simulink model "TosAeb"  (R2021a)
settings: Solver=FixedStepDiscrete  StartTime=0.0  StopTime=inf  FixedStep=0.01  RelTol=1e-3
undefined symbols (not defined in the file or in its folder): ACCModSts, AccSts1, AEB_ObjectMovingOrientation, AEB_ObjectMovingState, CN_BTCActive, CN_CurrentGearR, CN_CurrentGearR_DHT, CN_PTCActive, CN_VDCActive, CN_VSC_EspOff, CN_VSC_FCW_AEB_Error, CN_VSC_FCW_AEB_NoDispay, CN_VSC_FCW_AEB_PerfmDgdtn, CN_VSC_FCW_AEB_TempUnAvlbl, CN_VSC_VehStandstill, CNaccelAvoidMax, CNaccelAvoidMin, CNbrakePedalPress, CNcomfortStrLeftAccelMax, CNcomfortStrLeftAccelMin, CNcomfortStrRightAccelMax, CNcomfortStrRightAccelMin, CNDrvDoorOpen, CNDrvModeEco, CNDrvModeNorm, CNDrvModeOther, CNDrvModeSport, CNDrvSBRreminder, CNNull, CNobjInvalidID, CNPassDoorOpen, CNPassSBRreminder, CNPEBSAccelNegInf, CNPEBSAccelNull, CNPEBSTimeInf, CNPEBSTimeNull, CNSTOPMAX, CNSTOPNULL, CNsubjWidth, CNSwtOFF, CNSwtON, CNTTCMAX, CNTTCNULL, CNVehConfigAEBCycNone, CNVehConfigAEBNone, CNVehConfigAEBPedNone, CNVehConfigFCWNone, CNVehConfigJANone, CollisionSeverity, FALSE, FCWSnvty, JACycObjCalcInfoDefaultParams, JAPedObjCalcInfoDefaultParams, JAVehObjCalcInfoDefaultParams, latCycObjCalcInfoDefaultParams, latPedObjCalcInfoDefaultParams, longObjCalcInfoDefaultParams, ModSts, movingStatus, ObjectType ... (+1112)
libraries: simulink x3031, AEBSFunctionLib x50, Ta_Library x33, AEBSStateMachineLib x23, Mylibrary x12, simulink_extras x7, ObjSelect_lib x3, dspstat3 x2
(part 73 of 102)

== system TosAeb/FcwAebJa_Step_sys/ALG_AEBSystem/ALG_AEBController/AEB_LongTgtInfoCalc/LongObjCalcInfoCalc/AEBS_LongCollisionAssesApp/TTCCalc/TTCCalc/Situation2/distCorrCalc/AccelEqualZeroJudge ==
  subjLongAccel : Inport
  Abs : Abs
  Compare To Constant : simulink/Logic and Bit Operations/Compare To Constant  relop=<=  const=0.1  OutDataTypeStr=boolean
  accelEqualZeroFlg : Outport
  wires:
  subjLongAccel/1 -> Abs/1
  Compare To Constant/1 -> accelEqualZeroFlg/1
  Abs/1 -> Compare To Constant/1

== system TosAeb/FcwAebJa_Step_sys/ALG_AEBSystem/ALG_AEBController/AEB_LongTgtInfoCalc/LongObjCalcInfoCalc/AEBS_LongCollisionAssesApp/TTCCalc/TTCCalc/Situation2/distCorrCalc/distCorrCalc ==
  distEgo : Inport
  dxFrontBumper : Inport  Port=2
  Add : Sum
  distCorr : Outport
  wires:
  distEgo/1 -> Add/1
  dxFrontBumper/1 -> Add/2
  Add/1 -> distCorr/1

== system TosAeb/FcwAebJa_Step_sys/ALG_AEBSystem/ALG_AEBController/AEB_LongTgtInfoCalc/LongObjCalcInfoCalc/AEBS_LongCollisionAssesApp/TTCCalc/TTCCalc/Situation2/distCorrCalc/subjLongAccelEqualZero ==
  Action Port : ActionPort  ActionPortLabel=if(u1)
  Constant : Constant  Value=CNNull
  distEgo : Outport
  wires:
  Constant/1 -> distEgo/1

== system TosAeb/FcwAebJa_Step_sys/ALG_AEBSystem/ALG_AEBController/AEB_LongTgtInfoCalc/LongObjCalcInfoCalc/AEBS_LongCollisionAssesApp/TTCCalc/TTCCalc/Situation2/distCorrCalc/subjLongAccelNoEqualZero ==
  subjVel : Inport
  subjLongAccel : Inport  Port=2
  Action Port : ActionPort  ActionPortLabel=else
  Divide : Product  Inputs=*/
  Gain : Gain  Gain=0.5
  Math Function : Math  Operator=square  SignedPower=on  SaturateOnIntegerOverflow=on
  distEgo : Outport
  wires:
  subjVel/1 -> Math Function/1
  Math Function/1 -> Divide/1
  subjLongAccel/1 -> Divide/2
  Divide/1 -> Gain/1
  Gain/1 -> distEgo/1

== system TosAeb/FcwAebJa_Step_sys/ALG_AEBSystem/ALG_AEBController/AEB_LongTgtInfoCalc/LongObjCalcInfoCalc/AEBS_LongCollisionAssesApp/TTCCalc/TTCCalc/Situation3/SloveTTCQuadraticEquation/AccelRelEqualsZero ==
  distRel : Inport
  velRel : Inport  Port=2
  AccelRelEqualZero : ActionPort  ActionPortLabel=if(u1)
  Chart : Stateflow chart  TreatAsAtomicUnit=on
  TTC : Outport
  wires:
  Chart/1(TTC) -> TTC/1  "TTC"
  distRel/1 -> Chart/1(distRel)  "distRel"
  velRel/1 -> Chart/2(velRel)  "velRel"

== system TosAeb/FcwAebJa_Step_sys/ALG_AEBSystem/ALG_AEBController/AEB_LongTgtInfoCalc/LongObjCalcInfoCalc/AEBS_LongCollisionAssesApp/TTCCalc/TTCCalc/Situation3/SloveTTCQuadraticEquation/AccelRelUnequalZero ==
  accelRel : Inport
  velRel : Inport  Port=2
  distRel : Inport  Port=3
  AccelRelUnequalZero : ActionPort  ActionPortLabel=else
  DeltaCalcu : Subsystem
  DeltaGreaterEqualZero : Subsystem  TreatAsAtomicUnit=on
  DeltaSmallerZero : Subsystem  TreatAsAtomicUnit=on
  JudeDelta : If  IfExpression=u1 >= 0
  Merge : Merge
  TTC : Outport
  wires:
  Merge/1 -> TTC/1  "TTC"
  DeltaCalcu/1(delta) -> JudeDelta/1, DeltaGreaterEqualZero/1(delta)  "delta"
  accelRel/1 -> DeltaCalcu/1(accelRel), DeltaGreaterEqualZero/3(accelRel)  "accelRel"
  velRel/1 -> DeltaCalcu/2(velRel), DeltaGreaterEqualZero/2(velRel)  "velRel"
  distRel/1 -> DeltaCalcu/3(distRel)  "distRel"
  DeltaSmallerZero/1(TTC) -> Merge/2
  DeltaGreaterEqualZero/1(TTC) -> Merge/1
  JudeDelta/1 -> DeltaGreaterEqualZero/Action
  JudeDelta/2 -> [DeltaSmallZero]
  [DeltaSmallZero] -> DeltaSmallerZero/Action

== system TosAeb/FcwAebJa_Step_sys/ALG_AEBSystem/ALG_AEBController/AEB_LongTgtInfoCalc/LongObjCalcInfoCalc/AEBS_LongCollisionAssesApp/TTCCalc/TTCCalc/Situation3/SloveTTCQuadraticEquation/accelZeroFlgCalc ==
  accel : Inport
  Abs : Abs
  P_LongCA_AccelObjFiltThesTTC : simulink/Logic and Bit Operations/Compare To Constant  relop=<=  const=P_AEB_LongCA_AccelObjFiltThesTTC  OutDataTypeStr=boolean
  accelZeroFlg : Outport
  wires:
  Abs/1 -> P_LongCA_AccelObjFiltThesTTC/1
  P_LongCA_AccelObjFiltThesTTC/1 -> accelZeroFlg/1  "accelZeroFlg"
  accel/1 -> Abs/1  "accel"

== system TosAeb/FcwAebJa_Step_sys/ALG_AEBSystem/ALG_AEBController/AEB_LongTgtInfoCalc/LongObjCalcInfoCalc/AEBS_LongCollisionAssesApp/TTCCalc/TTCCalc/Situation3/distCorrCalc/AccelZeroJudge ==
  axAbsObj : Inport
  Abs : Abs
  Compare To Constant : simulink/Logic and Bit Operations/Compare To Constant  relop=<=  const=0.1  OutDataTypeStr=boolean
  accelZeroFlg : Outport
  wires:
  axAbsObj/1 -> Abs/1
  Compare To Constant/1 -> accelZeroFlg/1
  Abs/1 -> Compare To Constant/1

== system TosAeb/FcwAebJa_Step_sys/ALG_AEBSystem/ALG_AEBController/AEB_LongTgtInfoCalc/LongObjCalcInfoCalc/AEBS_LongCollisionAssesApp/TTCCalc/TTCCalc/Situation3/distCorrCalc/AxObjEqualZero ==
  Action Port : ActionPort  ActionPortLabel=if(u1)
  Constant : Constant  Value=CNNull
  distObj : Outport
  wires:
  Constant/1 -> distObj/1

== system TosAeb/FcwAebJa_Step_sys/ALG_AEBSystem/ALG_AEBController/AEB_LongTgtInfoCalc/LongObjCalcInfoCalc/AEBS_LongCollisionAssesApp/TTCCalc/TTCCalc/Situation3/distCorrCalc/AxObjUnequalZero ==
  vxAbsObj : Inport
  axAbsObj : Inport  Port=2
  Action Port : ActionPort  ActionPortLabel=else
  Divide : Product  Inputs=*/
  Gain : Gain  Gain=0.5
  Math Function : Math  Operator=square  SignedPower=on  SaturateOnIntegerOverflow=on
  distObj : Outport
  wires:
  Divide/1 -> Gain/1
  axAbsObj/1 -> Divide/2
  Math Function/1 -> Divide/1
  vxAbsObj/1 -> Math Function/1
  Gain/1 -> distObj/1

== system TosAeb/FcwAebJa_Step_sys/ALG_AEBSystem/ALG_AEBController/AEB_LongTgtInfoCalc/LongObjCalcInfoCalc/AEBS_LongCollisionAssesApp/TTCCalc/TTCCalc/Situation3/distCorrCalc/distCorrCalc ==
  dxFrontBumper : Inport
  distObj : Inport  Port=2
  Add : Sum  Inputs=+-
  distCorr : Outport
  wires:
  Add/1 -> distCorr/1
  dxFrontBumper/1 -> Add/1
  distObj/1 -> Add/2

== system TosAeb/FcwAebJa_Step_sys/ALG_AEBSystem/ALG_AEBController/AEB_LongTgtInfoCalc/LongObjCalcInfoCalc/AEBS_LongCollisionAssesApp/TTCCalc/TTCCalc/SloveTTCQuadraticEquation/AccelRelEqualsZero/Chart/TTCCalc ==
  distRel : Inport
  velRel : Inport  Port=2
  TTCCalc : TriggerPort  TriggerType=function-call  VariantControl=Variant
      settings: CopyFcn=Stateflow.SLINSF.SimulinkMan.removeBlockPreDeleteFcn  PreDeleteFcn=Stateflow.SLINSF.SimulinkMan.unsafePredeleteFcn
  Abs : Abs
  Abs1 : Abs
  Divide : Product  Inputs=*/
  tempTTC : Outport
  wires:
  Abs1/1 -> Divide/1
  Divide/1 -> tempTTC/1  "TTC"
  velRel/1 -> Abs/1  "velRel"
  distRel/1 -> Abs1/1  "distRel"
  Abs/1 -> Divide/2

== system TosAeb/FcwAebJa_Step_sys/ALG_AEBSystem/ALG_AEBController/AEB_LongTgtInfoCalc/LongObjCalcInfoCalc/AEBS_LongCollisionAssesApp/TTCCalc/TTCCalc/SloveTTCQuadraticEquation/AccelRelUnequalZero/DeltaCalcu ==
  accelRel : Inport
  velRel : Inport  Port=2
  distRel : Inport  Port=3
  Gain : Gain  Gain=2
  Math Function : Math  Operator=square  SignedPower=on  SaturateOnIntegerOverflow=on
  Product : Product
  Subtract : Sum  Inputs=+-
  delta : Outport
  wires:
  distRel/1 -> Product/2  "distRel"
  Math Function/1 -> Subtract/1
  Subtract/1 -> delta/1  "delta"
  Gain/1 -> Subtract/2
  Product/1 -> Gain/1
  velRel/1 -> Math Function/1  "velRel"
  accelRel/1 -> Product/1  "accelRel"

== system TosAeb/FcwAebJa_Step_sys/ALG_AEBSystem/ALG_AEBController/AEB_LongTgtInfoCalc/LongObjCalcInfoCalc/AEBS_LongCollisionAssesApp/TTCCalc/TTCCalc/SloveTTCQuadraticEquation/AccelRelUnequalZero/DeltaGreaterEqualZero ==
  delta : Inport
  velRel : Inport  Port=2
  accelRel : Inport  Port=3
  DeltaGreaterEqualZero : ActionPort  ActionPortLabel=if(u1 >= 0)
  Divide : Product  Inputs=*/
  Divide1 : Product  Inputs=*/
  Gain1 : Gain  Gain=-1
  RawTTCCalcu : Stateflow chart  TreatAsAtomicUnit=on
  Sqrt : Sqrt  SaturateOnIntegerOverflow=on
  Subtract1 : Sum  Inputs=+-
  Subtract2 : Sum
  TTC : Outport
  wires:
  Subtract1/1 -> Divide/1
  velRel/1 -> Gain1/1  "velRel"
  Subtract2/1 -> Divide1/1
  delta/1 -> Sqrt/1  "delta"
  Gain1/1 -> Subtract2/2, Subtract1/1
  accelRel/1 -> Divide/2, Divide1/2  "accelRel"
  Divide/1 -> RawTTCCalcu/1(TTC1)  "TTC1"
  Divide1/1 -> RawTTCCalcu/2(TTC2)  "TTC2"
  RawTTCCalcu/1(TTC) -> TTC/1  "TTC"
  Sqrt/1 -> Subtract2/1, Subtract1/2

== system TosAeb/FcwAebJa_Step_sys/ALG_AEBSystem/ALG_AEBController/AEB_LongTgtInfoCalc/LongObjCalcInfoCalc/AEBS_LongCollisionAssesApp/TTCCalc/TTCCalc/SloveTTCQuadraticEquation/AccelRelUnequalZero/DeltaSmallerZero ==
  DeltaSmallerZero : ActionPort  ActionPortLabel=else
  Constant : Constant  Value=CNTTCMAX
  TTC : Outport
  wires:
  Constant/1 -> TTC/1  "TTC"

== system TosAeb/FcwAebJa_Step_sys/ALG_AEBSystem/ALG_AEBController/AEB_LongTgtInfoCalc/LongObjCalcInfoCalc/AEBS_LongCollisionAssesApp/collisionSeverityHighCalc/collisionSeverityHighCalc1/CollisionAssessHighCalcVersion3/CollisionAssessHighCalc1/CalcBrakePhase1 ==
  a0Ego : Inport
  a0Obj : Inport  Port=2
  tBrakeLatency : Inport  Port=3
  velMaxReudce : Inport  Port=4
  v0Ego : Inport  Port=5
  v0Obj : Inport  Port=6
  dx : Inport  Port=7
  Bus Creator : BusCreator  Inputs=7
  Bus Selector : BusSelector  OutputSignals=a0Ego,v0Ego
  Bus Selector1 : BusSelector  OutputSignals=v0Ego,velMaxReudce,a0Ego,tBrakeLatency
  Bus Selector2 : BusSelector  OutputSignals=a0Obj,v0Obj
  Bus Selector3 : BusSelector  OutputSignals=v0Ego,a0Ego
  Bus Selector4 : BusSelector  OutputSignals=v0Obj,a0Obj
  Bus Selector5 : BusSelector  OutputSignals=v0Ego,a0Ego
  Bus Selector6 : BusSelector  OutputSignals=a0Obj,v0Obj
  Bus Selector7 : BusSelector  OutputSignals=dx
  Bus Selector8 : BusSelector  OutputSignals=velMaxReudce,v0Ego
  egoCollisionFlgCalc : Subsystem
  restDeltaVelCalc : Subsystem
  stEgoCalc : Subsystem
  stObjCalc : Subsystem
  tEgoStopCalc : Subsystem
  tObjRunCalc : Subsystem
  tObjStopCalc : Subsystem
  time4EgoPredic : Subsystem
  timeEgovDeltaCalc : Subsystem
  vtEgoCalc : Subsystem
  vtObjCalc : Subsystem
  egoCollisionFlg : Outport
  restDeltaVel : Outport  Port=2
  vtEgo : Outport  Port=3
  vtObj : Outport  Port=4
  dxt : Outport  Port=5
  wires:
  Bus Selector2/1 -> tObjStopCalc/1(a0Obj)  "<a0Obj>"
  Bus Selector2/2 -> tObjStopCalc/2(v0Obj)  "<v0Obj>"
  tEgoStopCalc/1(tEgoStop) -> time4EgoPredic/1(tEgoStop)
  Bus Selector/1 -> tEgoStopCalc/1(a0Ego)  "<a0Ego>"
  Bus Selector/2 -> tEgoStopCalc/2(v0Ego)  "<v0Ego>"
  Bus Creator/1 -> [BrakePhaseInput], Bus Selector2/1, Bus Selector1/1, Bus Selector/1, Bus Selector8/1, Bus Selector3/1
  Bus Selector6/2 -> stObjCalc/3(v0)  "<v0Obj>"
  Bus Selector6/1 -> stObjCalc/2(a0)  "<a0Obj>"
  v0Obj/1 -> Bus Creator/6  "v0Obj"
  v0Ego/1 -> Bus Creator/5  "v0Ego"
  velMaxReudce/1 -> Bus Creator/4  "velMaxReudce"
  tBrakeLatency/1 -> Bus Creator/3  "tBrakeLatency"
  a0Obj/1 -> Bus Creator/2  "a0Obj"
  dx/1 -> Bus Creator/7  "dx"
  timeEgovDeltaCalc/1(tEgovDelata) -> time4EgoPredic/2(tEgovDelata)
  time4EgoPredic/1(tEgoRun) -> vtEgoCalc/3(tTime), tObjRunCalc/1(tEgoRun), stEgoCalc/1(tTime)
  tObjStopCalc/1(tObjStop) -> tObjRunCalc/2(tObjStop)
  tObjRunCalc/1(tObjRun) -> stObjCalc/1(tTime), vtObjCalc/3(tTime)
  stEgoCalc/1(st) -> egoCollisionFlgCalc/1(stEgo)
  stObjCalc/1(st) -> egoCollisionFlgCalc/2(stObj)
  Bus Selector1/1 -> timeEgovDeltaCalc/1(v0Ego)  "<v0Ego>"
  Bus Selector1/2 -> timeEgovDeltaCalc/2(velMaxReudce)  "<velMaxReudce>"
  Bus Selector1/3 -> timeEgovDeltaCalc/3(a0Ego)  "<a0Ego>"
  Bus Selector1/4 -> time4EgoPredic/3(tBrakeLatency)  "<tBrakeLatency>"
  Bus Selector3/1 -> vtEgoCalc/1(v0)  "<v0Ego>"
  Bus Selector3/2 -> vtEgoCalc/2(a0)  "<a0Ego>"
  vtEgoCalc/1(vt) -> restDeltaVelCalc/3(vt), vtEgo/1
  vtObjCalc/1(vt) -> vtObj/1
  egoCollisionFlgCalc/2(egoCollisionFlg) -> egoCollisionFlg/1
  [BrakePhaseInput] -> Bus Selector4/1
  Bus Selector4/2 -> vtObjCalc/2(a0)  "<a0Obj>"
  Bus Selector4/1 -> vtObjCalc/1(v0)  "<v0Obj>"
  [BrakePhaseInput] -> Bus Selector5/1
  Bus Selector5/1 -> stEgoCalc/2(v0)  "<v0Ego>"
  Bus Selector5/2 -> stEgoCalc/3(a0)  "<a0Ego>"
  [BrakePhaseInput] -> Bus Selector6/1
  [BrakePhaseInput] -> Bus Selector7/1
  Bus Selector7/1 -> egoCollisionFlgCalc/3(dx)  "<dx>"
  a0Ego/1 -> Bus Creator/1  "a0Ego"
  Bus Selector8/1 -> restDeltaVelCalc/1(velMaxReudce)  "<velMaxReudce>"
  Bus Selector8/2 -> restDeltaVelCalc/2(v0)  "<v0Ego>"
  restDeltaVelCalc/1(restDeltaVel) -> restDeltaVel/1
  egoCollisionFlgCalc/1(dxt) -> dxt/1

== system TosAeb/FcwAebJa_Step_sys/ALG_AEBSystem/ALG_AEBController/AEB_LongTgtInfoCalc/LongObjCalcInfoCalc/AEBS_LongCollisionAssesApp/collisionSeverityHighCalc/collisionSeverityHighCalc1/CollisionAssessHighCalcVersion3/CollisionAssessHighCalc1/CalcBrakePhase2 ==
  accelMaxDec : Inport
  a0Ego : Inport  Port=2
  accelGradient : Inport  Port=3
  a0Obj : Inport  Port=4
  velMaxReudce : Inport  Port=5
  v0Ego : Inport  Port=6
  v0Obj : Inport  Port=7
  dx : Inport  Port=8
  BrkSysRuntimeFhase2 : Subsystem
  Bus Creator : BusCreator  Inputs=8
  Bus Selector : BusSelector  OutputSignals=a0Ego,v0Ego
  Bus Selector1 : BusSelector  OutputSignals=v0Ego,velMaxReudce,a0Ego
  Bus Selector2 : BusSelector  OutputSignals=a0Obj,v0Obj
  Bus Selector3 : BusSelector  OutputSignals=v0Ego,a0Ego,accelGradient
  Bus Selector4 : BusSelector  OutputSignals=v0Obj,a0Obj
  Bus Selector5 : BusSelector  OutputSignals=v0Ego,a0Ego,accelGradient
  Bus Selector6 : BusSelector  OutputSignals=a0Obj,v0Obj
  Bus Selector7 : BusSelector  OutputSignals=dx
  Bus Selector8 : BusSelector  OutputSignals=accelMaxDec,a0Ego,accelGradient
  Bus Selector9 : BusSelector  OutputSignals=velMaxReudce,v0Ego
  Subsystem1 : Subsystem
  egoCollisionFlgCalc : Subsystem
  restDeltaVelCalc : Subsystem
  stEgoCalc : Subsystem
  stObjCalc : Subsystem
  tEgoStopCalc : Subsystem
  tObjRunCalc : Subsystem
  tObjStopCalc : Subsystem
  time4EgoPredic : Subsystem
  timeEgovDeltaCalc : Subsystem
  vtObjCalc : Subsystem
  egoCollisionFlg : Outport
  restDeltaVelocity : Outport  Port=2
  vtEgo : Outport  Port=3
  vtObj : Outport  Port=4
  dxt : Outport  Port=5
  wires:
  accelMaxDec/1 -> Bus Creator/1  "accelMaxDec"
  Bus Selector/2 -> tEgoStopCalc/2(v0Ego)  "<v0Ego>"
  Bus Selector/1 -> tEgoStopCalc/1(a0Ego)  "<a0Ego>"
  Bus Selector2/2 -> tObjStopCalc/2(v0Obj)  "<v0Obj>"
  Bus Selector2/1 -> tObjStopCalc/1(a0Obj)  "<a0Obj>"
  egoCollisionFlgCalc/2(egoCollisionFlg) -> egoCollisionFlg/1
  Bus Selector1/1 -> timeEgovDeltaCalc/1(v0Ego)  "<v0Ego>"
  Bus Selector1/2 -> timeEgovDeltaCalc/2(velMaxReudce)  "<velMaxReudce>"
  Bus Selector1/3 -> timeEgovDeltaCalc/3(a0Ego)  "<a0Ego>"
  Bus Creator/1 -> [BrakePhaseInput], Bus Selector2/1, Bus Selector8/1, Bus Selector1/1, Bus Selector/1, Bus Selector3/1, Bus Selector9/1
  Bus Selector4/2 -> vtObjCalc/2(a0)  "<a0Obj>"
  [BrakePhaseInput] -> Bus Selector6/1
  [BrakePhaseInput] -> Bus Selector4/1
  tObjRunCalc/1(tObjRun) -> stObjCalc/1(tTime), vtObjCalc/3(tTime)
  Bus Selector4/1 -> vtObjCalc/1(v0)  "<v0Obj>"
  Bus Selector6/1 -> stObjCalc/2(a0)  "<a0Obj>"
  Bus Selector6/2 -> stObjCalc/3(v0)  "<v0Obj>"
  Bus Selector7/1 -> egoCollisionFlgCalc/3(dx)  "<dx>"
  [BrakePhaseInput] -> Bus Selector7/1
  Bus Selector5/3 -> stEgoCalc/4(accelGradient)  "<accelGradient>"
  Bus Selector5/2 -> stEgoCalc/3(a0)  "<a0Ego>"
  Bus Selector5/1 -> stEgoCalc/2(v0)  "<v0Ego>"
  [BrakePhaseInput] -> Bus Selector5/1
  vtObjCalc/1(vt) -> vtObj/1
  Subsystem1/1(vt) -> restDeltaVelCalc/3(vtEgo), vtEgo/1
  Bus Selector3/3 -> Subsystem1/3(accelGradient)  "<accelGradient>"
  Bus Selector3/2 -> Subsystem1/2(a0)  "<a0Ego>"
  Bus Selector3/1 -> Subsystem1/1(v0)  "<v0Ego>"
  stObjCalc/1(st) -> egoCollisionFlgCalc/2(stObj)
  stEgoCalc/1(st) -> egoCollisionFlgCalc/1(stEgo)
  tObjStopCalc/1(tObjStop) -> tObjRunCalc/2(tObjStop)
  time4EgoPredic/1(tEgoRun) -> stEgoCalc/1(tTime), tObjRunCalc/1(tEgoRun), Subsystem1/4(tTime)
  timeEgovDeltaCalc/1(tEgovDelata) -> time4EgoPredic/2(tEgovDelata)
  tEgoStopCalc/1(tEgoStop) -> time4EgoPredic/1(tEgoStop)
  dx/1 -> Bus Creator/8  "dx"
  a0Ego/1 -> Bus Creator/2  "a0Ego"
  accelGradient/1 -> Bus Creator/3  "accelGradient"
  a0Obj/1 -> Bus Creator/4  "a0Obj"
  velMaxReudce/1 -> Bus Creator/5  "velMaxReudce"
  v0Ego/1 -> Bus Creator/6  "v0Ego"
  v0Obj/1 -> Bus Creator/7  "v0Obj"
  BrkSysRuntimeFhase2/1(tTime) -> time4EgoPredic/3(tTime)
  Bus Selector8/1 -> BrkSysRuntimeFhase2/1(accelMaxDec)  "<accelMaxDec>"
  Bus Selector8/2 -> BrkSysRuntimeFhase2/2(a0Ego)  "<a0Ego>"
  Bus Selector8/3 -> BrkSysRuntimeFhase2/3(accelGradient)  "<accelGradient>"
  Bus Selector9/2 -> restDeltaVelCalc/2(v0Ego)  "<v0Ego>"
  Bus Selector9/1 -> restDeltaVelCalc/1(velMaxReudce)  "<velMaxReudce>"
  restDeltaVelCalc/1(restDeltaVelocity) -> restDeltaVelocity/1
  egoCollisionFlgCalc/1(dxt) -> dxt/1

== system TosAeb/FcwAebJa_Step_sys/ALG_AEBSystem/ALG_AEBController/AEB_LongTgtInfoCalc/LongObjCalcInfoCalc/AEBS_LongCollisionAssesApp/collisionSeverityHighCalc/collisionSeverityHighCalc1/CollisionAssessHighCalcVersion3/CollisionAssessHighCalc1/CalcBrakePhase3 ==
  a0Ego : Inport
  a0Obj : Inport  Port=2
  accelMaxDec : Inport  Port=3
  velMaxReudce : Inport  Port=4
  v0Ego : Inport  Port=5
  v0Obj : Inport  Port=6
  dx0 : Inport  Port=7
  BrkSysRuntime : Subsystem
  Bus Creator : BusCreator  Inputs=7
  Bus Selector : BusSelector  OutputSignals=a0Ego,v0Ego
  Bus Selector1 : BusSelector  OutputSignals=v0Ego,velMaxReudce,a0Ego
  Bus Selector2 : BusSelector  OutputSignals=a0Obj,v0Obj
  Bus Selector5 : BusSelector  OutputSignals=v0Ego,a0Ego
  Bus Selector6 : BusSelector  OutputSignals=a0Obj,v0Obj
  Bus Selector7 : BusSelector  OutputSignals=dx0
  Bus Selector8 : BusSelector  OutputSignals=a0Obj,accelMaxDec,v0Obj,v0Ego
  egoCollisionFlgCalc : Subsystem
  stEgoCalc : Subsystem
  stObjCalc : Subsystem
  tEgoStopCalc : Subsystem
  tObjRunCalc : Subsystem
  tObjStopCalc : Subsystem
  time4EgoPredic : Subsystem
  timeEgovDeltaCalc : Subsystem
  egoCollisionFlg : Outport
  wires:
  accelMaxDec/1 -> Bus Creator/3  "accelMaxDec"
  Bus Selector/2 -> tEgoStopCalc/2(v0Ego)  "<v0Ego>"
  Bus Selector/1 -> tEgoStopCalc/1(a0Ego)  "<a0Ego>"
  Bus Selector2/2 -> tObjStopCalc/2(v0Obj)  "<v0Obj>"
  Bus Selector2/1 -> tObjStopCalc/1(a0Obj)  "<a0Obj>"
  [BrakePhaseInput] -> Bus Selector6/1
  Bus Selector6/1 -> stObjCalc/2(a0)  "<a0Obj>"
  Bus Selector6/2 -> stObjCalc/3(v0)  "<v0Obj>"
  tObjRunCalc/1(tObjRun) -> stObjCalc/1(tTime)
  stObjCalc/1(st) -> egoCollisionFlgCalc/2(stObj)
  tEgoStopCalc/1(tEgoStop) -> time4EgoPredic/1(tEgoStop)
  timeEgovDeltaCalc/1(tEgovDelata) -> time4EgoPredic/2(tEgovDelata)
  Bus Selector1/1 -> timeEgovDeltaCalc/1(v0Ego)  "<v0Ego>"
  Bus Selector1/3 -> timeEgovDeltaCalc/3(a0Ego)  "<a0Ego>"
  Bus Selector1/2 -> timeEgovDeltaCalc/2(velMaxReudce)  "<velMaxReudce>"
  Bus Creator/1 -> [BrakePhaseInput], Bus Selector2/1, Bus Selector8/1, Bus Selector1/1, Bus Selector/1
  v0Obj/1 -> Bus Creator/6  "v0Obj"
  v0Ego/1 -> Bus Creator/5  "v0Ego"
  velMaxReudce/1 -> Bus Creator/4  "velMaxReudce"
  a0Obj/1 -> Bus Creator/2  "a0Obj"
  dx0/1 -> Bus Creator/7  "dx0"
  time4EgoPredic/1(tEgoRun) -> tObjRunCalc/1(tEgoRun), stEgoCalc/1(tTime)
  tObjStopCalc/1(tObjStop) -> tObjRunCalc/2(tObjStop)
  stEgoCalc/1(st) -> egoCollisionFlgCalc/1(stEgo)
  egoCollisionFlgCalc/1(egoCollisionFlg) -> egoCollisionFlg/1
  [BrakePhaseInput] -> Bus Selector5/1
  Bus Selector5/1 -> stEgoCalc/2(v0)  "<v0Ego>"
  Bus Selector5/2 -> stEgoCalc/3(a0)  "<a0Ego>"
  [BrakePhaseInput] -> Bus Selector7/1
  Bus Selector7/1 -> egoCollisionFlgCalc/3(dx0)  "<dx0>"
  a0Ego/1 -> Bus Creator/1  "a0Ego"
  Bus Selector8/1 -> BrkSysRuntime/1(a0Obj)  "<a0Obj>"
  Bus Selector8/2 -> BrkSysRuntime/2(accelMaxDec)  "<accelMaxDec>"
  Bus Selector8/3 -> BrkSysRuntime/3(v0Obj)  "<v0Obj>"
  Bus Selector8/4 -> BrkSysRuntime/4(v0Ego)  "<v0Ego>"
  BrkSysRuntime/1(tTime) -> time4EgoPredic/3(tTime)

== system TosAeb/FcwAebJa_Step_sys/ALG_AEBSystem/ALG_AEBController/AEB_LongTgtInfoCalc/LongObjCalcInfoCalc/AEBS_LongCollisionAssesApp/collisionSeverityHighCalc/collisionSeverityHighCalc1/CollisionAssessHighCalcVersion3/CollisionAssessHighCalc1/collisionSeverityHighFlgJudge ==
  egoCollisionFlgPhase1 : Inport
  egoCollisionFlgPhase2 : Inport  Port=2
  egoCollisionFlgPhase3 : Inport  Port=3
  Logical Operator : Logic  Operator=OR  Inputs=3  AllPortsSameDT=off  OutDataTypeStr=boolean
  collisionSeverityHighFlg : Outport
  wires:
  egoCollisionFlgPhase2/1 -> Logical Operator/2
  egoCollisionFlgPhase1/1 -> Logical Operator/1
  egoCollisionFlgPhase3/1 -> Logical Operator/3
  Logical Operator/1 -> collisionSeverityHighFlg/1

== system TosAeb/FcwAebJa_Step_sys/ALG_AEBSystem/ALG_AEBController/AEB_LongTgtInfoCalc/LongObjCalcInfoCalc/AEBS_LongCollisionAssesApp/collisionSeverityHighCalc/collisionSeverityHighCalc1/CollisionAssessHighCalcVersion3/CollisionAssessHighCalc1/dxWithoutSecureCalc ==
  dxFrontBumper : Inport
  dxSecure : Inport  Port=2
  Compare To Constant : simulink/Logic and Bit Operations/Compare To Constant  relop=<  const=0  OutDataTypeStr=boolean
  Constant : Constant  Value=0
  Subtract : Sum  Inputs=+-
  Switch : Switch  Criteria=u2 ~= 0
  dxWithoutSecure : Outport
  wires:
  Subtract/1 -> Switch/3, Compare To Constant/1
  Compare To Constant/1 -> Switch/2
  Switch/1 -> dxWithoutSecure/1
  Constant/1 -> Switch/1
  dxFrontBumper/1 -> Subtract/1
  dxSecure/1 -> Subtract/2

== system TosAeb/FcwAebJa_Step_sys/ALG_AEBSystem/ALG_AEBController/AEB_LongTgtInfoCalc/LongObjCalcInfoCalc/AEBS_LongCollisionAssesApp/collisionSeverityHighCalc/collisionSeverityHighCalc1/CollisionAssessHighCalcVersion3/CollisionAssessHighCalc1/subjLongAccelFiltCalc ==
  subjLongAccel : Inport
  Compare To Constant : simulink/Logic and Bit Operations/Compare To Constant  relop=>  const=CNNull  OutDataTypeStr=boolean
  Constant : Constant  Value=CNNull
  Switch : Switch  Criteria=u2 ~= 0
  subjLongAccelFilt : Outport
  wires:
  Compare To Constant/1 -> Switch/2
  subjLongAccel/1 -> Switch/3, Compare To Constant/1
  Constant/1 -> Switch/1
  Switch/1 -> subjLongAccelFilt/1

== system TosAeb/FcwAebJa_Step_sys/ALG_AEBSystem/ALG_AEBController/AEB_LongTgtInfoCalc/LongObjCalcInfoCalc/AEBS_LongCollisionAssesApp/collisionSeverityHighCalc/collisionSeverityHighCalc1/CollisionAssessHighCalcVersion3/CollisionAssessHighCalc2/CalcBrakePhase1 ==
  a0Ego : Inport
  a0Obj : Inport  Port=2
  tBrakeLatency : Inport  Port=3
  velMaxReudce : Inport  Port=4
  v0Ego : Inport  Port=5
  s0Ego : Inport  Port=6
  v0Obj : Inport  Port=7
  s0Obj : Inport  Port=8
  dx : Inport  Port=9
  Bus Creator : BusCreator  Inputs=9
  Bus Selector : BusSelector  OutputSignals=a0Ego,v0Ego
  Bus Selector1 : BusSelector  OutputSignals=v0Ego,velMaxReudce,a0Ego,tBrakeLatency
  Bus Selector2 : BusSelector  OutputSignals=a0Obj,v0Obj
  Bus Selector3 : BusSelector  OutputSignals=v0Ego,a0Ego
  Bus Selector4 : BusSelector  OutputSignals=v0Obj,a0Obj
  Bus Selector5 : BusSelector  OutputSignals=s0Ego,v0Ego,a0Ego
  Bus Selector6 : BusSelector  OutputSignals=a0Obj,v0Obj,s0Obj
  Bus Selector7 : BusSelector  OutputSignals=dx
  Bus Selector8 : BusSelector  OutputSignals=velMaxReudce,v0Ego
  egoCollisionFlgCalc : Subsystem
  restDeltaVelCalc : Subsystem
  stEgoCalc : Subsystem
  stObjCalc : Subsystem
  tEgoStopCalc : Subsystem
  tObjRunCalc : Subsystem
  tObjStopCalc : Subsystem
  time4EgoPredic : Subsystem
  timeEgovDelataCalc : Subsystem
  vtEgoCalc : Subsystem
  vtObjCalc : Subsystem
  egoCollisionFlg : Outport
  restDeltaVel : Outport  Port=2
  vtEgo : Outport  Port=3
  stEgo : Outport  Port=4
  vtObj : Outport  Port=5
  stObj : Outport  Port=6
  wires:
  Bus Selector2/1 -> tObjStopCalc/1(a0Obj)  "<a0Obj>"
  Bus Selector2/2 -> tObjStopCalc/2(v0Obj)  "<v0Obj>"
  tEgoStopCalc/1(tEgoStop) -> time4EgoPredic/1(tEgoStop)
  Bus Selector/1 -> tEgoStopCalc/1(a0Ego)  "<a0Ego>"
  Bus Selector/2 -> tEgoStopCalc/2(v0Ego)  "<v0Ego>"
  Bus Creator/1 -> [BrakePhaseInput], Bus Selector2/1, Bus Selector1/1, Bus Selector/1, Bus Selector8/1, Bus Selector3/1
  Bus Selector6/3 -> stObjCalc/4(s0)  "<s0Obj>"
  Bus Selector6/2 -> stObjCalc/3(v0)  "<v0Obj>"
  Bus Selector6/1 -> stObjCalc/2(a0)  "<a0Obj>"
  s0Obj/1 -> Bus Creator/8  "s0Obj"
  v0Obj/1 -> Bus Creator/7  "v0Obj"
  s0Ego/1 -> Bus Creator/6  "s0Ego"
  v0Ego/1 -> Bus Creator/5  "v0Ego"
  velMaxReudce/1 -> Bus Creator/4  "velMaxReudce"
  tBrakeLatency/1 -> Bus Creator/3  "tBrakeLatency"
  a0Obj/1 -> Bus Creator/2  "a0Obj"
  dx/1 -> Bus Creator/9  "dx"
  timeEgovDelataCalc/1(tEgovDelata) -> time4EgoPredic/2(tEgovDelata)
  time4EgoPredic/1(tEgoRun) -> vtEgoCalc/3(tTime), tObjRunCalc/1(tEgoRun), stEgoCalc/1(tTime)
  tObjStopCalc/1(tObjStop) -> tObjRunCalc/2(tObjStop)
  tObjRunCalc/1(tObjRun) -> stObjCalc/1(tTime), vtObjCalc/3(tTime)
  stEgoCalc/1(st) -> egoCollisionFlgCalc/1(stEgo), stEgo/1
  stObjCalc/1(st) -> stObj/1, egoCollisionFlgCalc/2(stObj)
  Bus Selector1/1 -> timeEgovDelataCalc/1(v0Ego)  "<v0Ego>"
  Bus Selector1/2 -> timeEgovDelataCalc/2(velMaxReudce)  "<velMaxReudce>"
  Bus Selector1/3 -> timeEgovDelataCalc/3(a0Ego)  "<a0Ego>"
  Bus Selector1/4 -> time4EgoPredic/3(tBrakeLatency)  "<tBrakeLatency>"
  Bus Selector3/1 -> vtEgoCalc/1(v0)  "<v0Ego>"
  Bus Selector3/2 -> vtEgoCalc/2(a0)  "<a0Ego>"
  vtEgoCalc/1(vt) -> restDeltaVelCalc/3(vt), vtEgo/1
  vtObjCalc/1(vt) -> vtObj/1
  egoCollisionFlgCalc/1(egoCollisionFlg) -> egoCollisionFlg/1
  [BrakePhaseInput] -> Bus Selector4/1
  Bus Selector4/2 -> vtObjCalc/2(a0)  "<a0Obj>"
  Bus Selector4/1 -> vtObjCalc/1(v0)  "<v0Obj>"
  [BrakePhaseInput] -> Bus Selector5/1
  Bus Selector5/1 -> stEgoCalc/2(s0)  "<s0Ego>"
  Bus Selector5/2 -> stEgoCalc/3(v0)  "<v0Ego>"
  Bus Selector5/3 -> stEgoCalc/4(a0)  "<a0Ego>"
  [BrakePhaseInput] -> Bus Selector6/1
  [BrakePhaseInput] -> Bus Selector7/1
  Bus Selector7/1 -> egoCollisionFlgCalc/3(dx)  "<dx>"
  a0Ego/1 -> Bus Creator/1  "a0Ego"
  Bus Selector8/1 -> restDeltaVelCalc/1(velMaxReudce)  "<velMaxReudce>"
  Bus Selector8/2 -> restDeltaVelCalc/2(v0)  "<v0Ego>"
  restDeltaVelCalc/1(restDeltaVel) -> restDeltaVel/1

== system TosAeb/FcwAebJa_Step_sys/ALG_AEBSystem/ALG_AEBController/AEB_LongTgtInfoCalc/LongObjCalcInfoCalc/AEBS_LongCollisionAssesApp/collisionSeverityHighCalc/collisionSeverityHighCalc1/CollisionAssessHighCalcVersion3/CollisionAssessHighCalc2/CalcBrakePhase2 ==
  accelMaxDec : Inport
  a0Ego : Inport  Port=2
  accelGradient : Inport  Port=3
  a0Obj : Inport  Port=4
  velMaxReudce : Inport  Port=5
  v0Ego : Inport  Port=6
  s0Ego : Inport  Port=7
  v0Obj : Inport  Port=8
  s0Obj : Inport  Port=9
  dx : Inport  Port=10
  BrkSysRuntimeFhase2 : Subsystem
  Bus Creator : BusCreator  Inputs=10
  Bus Selector : BusSelector  OutputSignals=a0Ego,v0Ego
  Bus Selector1 : BusSelector  OutputSignals=v0Ego,velMaxReudce,a0Ego
  Bus Selector2 : BusSelector  OutputSignals=a0Obj,v0Obj
  Bus Selector3 : BusSelector  OutputSignals=v0Ego,a0Ego,accelGradient
  Bus Selector4 : BusSelector  OutputSignals=v0Obj,a0Obj
  Bus Selector5 : BusSelector  OutputSignals=s0Ego,v0Ego,a0Ego,accelGradient
  Bus Selector6 : BusSelector  OutputSignals=a0Obj,v0Obj,s0Obj
  Bus Selector7 : BusSelector  OutputSignals=dx
  Bus Selector8 : BusSelector  OutputSignals=accelMaxDec,a0Ego,accelGradient
  Bus Selector9 : BusSelector  OutputSignals=velMaxReudce,v0Ego
  Subsystem1 : Subsystem
  egoCollisionFlgCalc : Subsystem
  restDeltaVelCalc : Subsystem
  stEgoCalc : Subsystem
  stObjCalc : Subsystem
  tEgoStopCalc : Subsystem
  tObjRunCalc : Subsystem
  tObjStopCalc : Subsystem
  time4EgoPredic : Subsystem
  timeEgovDelataCalc : Subsystem
  vtObjCalc : Subsystem
  egoCollisionFlg : Outport
  restDeltaVelocity : Outport  Port=2
  vtEgo : Outport  Port=3
  stEgo : Outport  Port=4
  vtObj : Outport  Port=5
  stObj : Outport  Port=6
  wires:
  accelMaxDec/1 -> Bus Creator/1  "accelMaxDec"
  Bus Selector/2 -> tEgoStopCalc/2(v0Ego)  "<v0Ego>"
  Bus Selector/1 -> tEgoStopCalc/1(a0Ego)  "<a0Ego>"
  Bus Selector2/2 -> tObjStopCalc/2(v0Obj)  "<v0Obj>"
  Bus Selector2/1 -> tObjStopCalc/1(a0Obj)  "<a0Obj>"
  egoCollisionFlgCalc/1(egoCollisionFlg) -> egoCollisionFlg/1
  Bus Selector1/1 -> timeEgovDelataCalc/1(v0Ego)  "<v0Ego>"
  Bus Selector1/2 -> timeEgovDelataCalc/2(velMaxReudce)  "<velMaxReudce>"
  Bus Selector1/3 -> timeEgovDelataCalc/3(a0Ego)  "<a0Ego>"
  Bus Creator/1 -> [BrakePhaseInput], Bus Selector2/1, Bus Selector8/1, Bus Selector1/1, Bus Selector/1, Bus Selector3/1, Bus Selector9/1
  Bus Selector6/3 -> stObjCalc/4(s0)  "<s0Obj>"
  Bus Selector4/2 -> vtObjCalc/2(a0)  "<a0Obj>"
  [BrakePhaseInput] -> Bus Selector6/1
  [BrakePhaseInput] -> Bus Selector4/1
  tObjRunCalc/1(tObjRun) -> stObjCalc/1(tTime), vtObjCalc/3(tTime)
  Bus Selector4/1 -> vtObjCalc/1(v0)  "<v0Obj>"
  Bus Selector6/1 -> stObjCalc/2(a0)  "<a0Obj>"
  Bus Selector6/2 -> stObjCalc/3(v0)  "<v0Obj>"
  Bus Selector7/1 -> egoCollisionFlgCalc/3(dx)  "<dx>"
  [BrakePhaseInput] -> Bus Selector7/1
  Bus Selector5/4 -> stEgoCalc/5(accelGradient)  "<accelGradient>"
  Bus Selector5/3 -> stEgoCalc/4(a0)  "<a0Ego>"
  Bus Selector5/2 -> stEgoCalc/3(v0)  "<v0Ego>"
  Bus Selector5/1 -> stEgoCalc/2(s0)  "<s0Ego>"
  [BrakePhaseInput] -> Bus Selector5/1
  vtObjCalc/1(vt) -> vtObj/1
  Subsystem1/1(vt) -> restDeltaVelCalc/3(vtEgo), vtEgo/1
  Bus Selector3/3 -> Subsystem1/3(accelGradient)  "<accelGradient>"
  Bus Selector3/2 -> Subsystem1/2(a0)  "<a0Ego>"
  Bus Selector3/1 -> Subsystem1/1(v0)  "<v0Ego>"
  stObjCalc/1(st) -> stObj/1, egoCollisionFlgCalc/2(stObj)
  stEgoCalc/1(st) -> stEgo/1, egoCollisionFlgCalc/1(stEgo)
  tObjStopCalc/1(tObjStop) -> tObjRunCalc/2(tObjStop)
  time4EgoPredic/1(tEgoRun) -> stEgoCalc/1(tTime), tObjRunCalc/1(tEgoRun), Subsystem1/4(tTime)
  timeEgovDelataCalc/1(tEgovDelata) -> time4EgoPredic/2(tEgovDelata)
  tEgoStopCalc/1(tEgoStop) -> time4EgoPredic/1(tEgoStop)
  dx/1 -> Bus Creator/10  "dx"
  a0Ego/1 -> Bus Creator/2  "a0Ego"
  accelGradient/1 -> Bus Creator/3  "accelGradient"
  a0Obj/1 -> Bus Creator/4  "a0Obj"
  velMaxReudce/1 -> Bus Creator/5  "velMaxReudce"
  v0Ego/1 -> Bus Creator/6  "v0Ego"
  s0Ego/1 -> Bus Creator/7  "s0Ego"
  v0Obj/1 -> Bus Creator/8  "v0Obj"
  s0Obj/1 -> Bus Creator/9  "s0Obj"
  BrkSysRuntimeFhase2/1(tTime) -> time4EgoPredic/3(tTime)
  Bus Selector8/1 -> BrkSysRuntimeFhase2/1(accelMaxDec)  "<accelMaxDec>"
  Bus Selector8/2 -> BrkSysRuntimeFhase2/2(a0Ego)  "<a0Ego>"
  Bus Selector8/3 -> BrkSysRuntimeFhase2/3(accelGradient)  "<accelGradient>"
  Bus Selector9/2 -> restDeltaVelCalc/2(v0Ego)  "<v0Ego>"
  Bus Selector9/1 -> restDeltaVelCalc/1(velMaxReudce)  "<velMaxReudce>"
  restDeltaVelCalc/1(restDeltaVelocity) -> restDeltaVelocity/1

== system TosAeb/FcwAebJa_Step_sys/ALG_AEBSystem/ALG_AEBController/AEB_LongTgtInfoCalc/LongObjCalcInfoCalc/AEBS_LongCollisionAssesApp/collisionSeverityHighCalc/collisionSeverityHighCalc1/CollisionAssessHighCalcVersion3/CollisionAssessHighCalc2/CalcBrakePhase3 ==
  a0Ego : Inport
  a0Obj : Inport  Port=2
  accelMaxDec : Inport  Port=3
  velMaxReudce : Inport  Port=4
  v0Ego : Inport  Port=5
  s0Ego : Inport  Port=6
  v0Obj : Inport  Port=7
  s0Obj : Inport  Port=8
  dx : Inport  Port=9
  BrkSysRuntime : Subsystem
  Bus Creator : BusCreator  Inputs=9
  Bus Selector : BusSelector  OutputSignals=a0Ego,v0Ego
  Bus Selector1 : BusSelector  OutputSignals=v0Ego,velMaxReudce,a0Ego
  Bus Selector2 : BusSelector  OutputSignals=a0Obj,v0Obj
  Bus Selector5 : BusSelector  OutputSignals=s0Ego,v0Ego,a0Ego
  Bus Selector6 : BusSelector  OutputSignals=a0Obj,v0Obj,s0Obj
  Bus Selector7 : BusSelector  OutputSignals=dx
  Bus Selector8 : BusSelector  OutputSignals=a0Obj,accelMaxDec,v0Obj,v0Ego
  egoCollisionFlgCalc : Subsystem
  stEgoCalc : Subsystem
  stObjCalc : Subsystem
  tEgoStopCalc : Subsystem
  tObjRunCalc : Subsystem
  tObjStopCalc : Subsystem
  time4EgoPredic : Subsystem
  timeEgovDelataCalc : Subsystem
  egoCollisionFlg : Outport
  wires:
  accelMaxDec/1 -> Bus Creator/3  "accelMaxDec"
  Bus Selector/2 -> tEgoStopCalc/2(v0Ego)  "<v0Ego>"
  Bus Selector/1 -> tEgoStopCalc/1(a0Ego)  "<a0Ego>"
  Bus Selector2/2 -> tObjStopCalc/2(v0Obj)  "<v0Obj>"
  Bus Selector2/1 -> tObjStopCalc/1(a0Obj)  "<a0Obj>"
  [BrakePhaseInput] -> Bus Selector6/1
  Bus Selector6/1 -> stObjCalc/2(a0)  "<a0Obj>"
  Bus Selector6/2 -> stObjCalc/3(v0)  "<v0Obj>"
  tObjRunCalc/1(tObjRun) -> stObjCalc/1(tTime)
  stObjCalc/1(st) -> egoCollisionFlgCalc/2(stObj)
  Bus Selector6/3 -> stObjCalc/4(s0)  "<s0Obj>"
  tEgoStopCalc/1(tEgoStop) -> time4EgoPredic/1(tEgoStop)
  timeEgovDelataCalc/1(tEgovDelata) -> time4EgoPredic/2(tEgovDelata)
  Bus Selector1/1 -> timeEgovDelataCalc/1(v0Ego)  "<v0Ego>"
  Bus Selector1/3 -> timeEgovDelataCalc/3(a0Ego)  "<a0Ego>"
  Bus Selector1/2 -> timeEgovDelataCalc/2(velMaxReudce)  "<velMaxReudce>"
  Bus Creator/1 -> [BrakePhaseInput], Bus Selector2/1, Bus Selector8/1, Bus Selector1/1, Bus Selector/1
  s0Obj/1 -> Bus Creator/8  "s0Obj"
  v0Obj/1 -> Bus Creator/7  "v0Obj"
  s0Ego/1 -> Bus Creator/6  "s0Ego"
  v0Ego/1 -> Bus Creator/5  "v0Ego"
  velMaxReudce/1 -> Bus Creator/4  "velMaxReudce"
  a0Obj/1 -> Bus Creator/2  "a0Obj"
  dx/1 -> Bus Creator/9  "dx"
  time4EgoPredic/1(tEgoRun) -> stEgoCalc/1(tTime), tObjRunCalc/1(tEgoRun)
  tObjStopCalc/1(tObjStop) -> tObjRunCalc/2(tObjStop)
  stEgoCalc/1(st) -> egoCollisionFlgCalc/1(stEgo)
  egoCollisionFlgCalc/1(egoCollisionFlg) -> egoCollisionFlg/1
  [BrakePhaseInput] -> Bus Selector5/1
  Bus Selector5/1 -> stEgoCalc/2(s0)  "<s0Ego>"
  Bus Selector5/2 -> stEgoCalc/3(v0)  "<v0Ego>"
  Bus Selector5/3 -> stEgoCalc/4(a0)  "<a0Ego>"
  [BrakePhaseInput] -> Bus Selector7/1
  Bus Selector7/1 -> egoCollisionFlgCalc/3(dx)  "<dx>"
  a0Ego/1 -> Bus Creator/1  "a0Ego"
  Bus Selector8/1 -> BrkSysRuntime/1(a0Obj)  "<a0Obj>"
  Bus Selector8/2 -> BrkSysRuntime/2(accelMaxDec)  "<accelMaxDec>"
  Bus Selector8/3 -> BrkSysRuntime/3(v0Obj)  "<v0Obj>"
  Bus Selector8/4 -> BrkSysRuntime/4(v0Ego)  "<v0Ego>"
  BrkSysRuntime/1(tTime) -> time4EgoPredic/3(tTime)

== system TosAeb/FcwAebJa_Step_sys/ALG_AEBSystem/ALG_AEBController/AEB_LongTgtInfoCalc/LongObjCalcInfoCalc/AEBS_LongCollisionAssesApp/collisionSeverityHighCalc/collisionSeverityHighCalc1/CollisionAssessHighCalcVersion3/CollisionAssessHighCalc2/collisionSeverityHighFlgJudge ==
  egoCollisionFlgPhase1 : Inport
  egoCollisionFlgPhase2 : Inport  Port=2
  egoCollisionFlgPhase3 : Inport  Port=3
  Logical Operator : Logic  Operator=OR  Inputs=3  AllPortsSameDT=off  OutDataTypeStr=boolean
  collisionSeverityHighFlg : Outport
  wires:
  egoCollisionFlgPhase2/1 -> Logical Operator/2
  egoCollisionFlgPhase1/1 -> Logical Operator/1
  egoCollisionFlgPhase3/1 -> Logical Operator/3
  Logical Operator/1 -> collisionSeverityHighFlg/1

== system TosAeb/FcwAebJa_Step_sys/ALG_AEBSystem/ALG_AEBController/AEB_LongTgtInfoCalc/LongObjCalcInfoCalc/AEBS_LongCollisionAssesApp/collisionSeverityHighCalc/collisionSeverityHighCalc1/CollisionAssessHighCalcVersion3/CollisionAssessHighCalc2/dxWithoutSecureCalc ==
  dxFrontBumper : Inport
  dxSecure : Inport  Port=2
  Compare To Constant : simulink/Logic and Bit Operations/Compare To Constant  relop=<  const=0  OutDataTypeStr=boolean
  Constant : Constant  Value=0
  Subtract : Sum  Inputs=+-
  Switch : Switch  Criteria=u2 ~= 0
  dxWithoutSecure : Outport
  wires:
  Subtract/1 -> Switch/3, Compare To Constant/1
  Compare To Constant/1 -> Switch/2
  Switch/1 -> dxWithoutSecure/1
  Constant/1 -> Switch/1
  dxFrontBumper/1 -> Subtract/1
  dxSecure/1 -> Subtract/2

== system TosAeb/FcwAebJa_Step_sys/ALG_AEBSystem/ALG_AEBController/AEB_LongTgtInfoCalc/LongObjCalcInfoCalc/AEBS_LongCollisionAssesApp/collisionSeverityHighCalc/collisionSeverityHighCalc1/CollisionAssessHighCalcVersion3/CollisionAssessHighCalc2/subjLongAccelFiltCalc ==
  subjLongAccel : Inport
  Compare To Constant : simulink/Logic and Bit Operations/Compare To Constant  relop=>  const=0  OutDataTypeStr=boolean
  Constant : Constant  Value=0
  Switch : Switch  Criteria=u2 ~= 0
  subjLongAccelFilt : Outport
  wires:
  Compare To Constant/1 -> Switch/2
  subjLongAccel/1 -> Switch/3, Compare To Constant/1
  Constant/1 -> Switch/1
  Switch/1 -> subjLongAccelFilt/1

== system TosAeb/FcwAebJa_Step_sys/ALG_AEBSystem/ALG_AEBController/AEB_LongTgtInfoCalc/LongObjCalcInfoCalc/AEBS_LongCollisionAssesApp/collisionSeverityHighCalc/collisionSeverityHighCalc2/collisionSeverityHighPhase1/Phase1TimeCalc/BrakeLatencyTimeCalc ==
  subjLongAccel : Inport
  DelayTimeHigh : Constant  Value=PcollisionDgrLvl3BrkDelayTimeHigh
  DelayTimeLow : Constant  Value=PcollisionDgrLvl3BrkDelayTimeLow
  SubjAccelThres : simulink/Logic and Bit Operations/Compare To Constant  relop=<  const=PcollisionDgrLvl3SubjAccelThes4BrkDelayTime  OutDataTypeStr=boolean
  Switch1 : Switch  Criteria=u2 ~= 0
  tBrakeLatency : Outport
  wires:
  DelayTimeLow/1 -> Switch1/1
  DelayTimeHigh/1 -> Switch1/3
  Switch1/1 -> tBrakeLatency/1  "tBrakeLatency"
  SubjAccelThres/1 -> Switch1/2
  subjLongAccel/1 -> SubjAccelThres/1

== system TosAeb/FcwAebJa_Step_sys/ALG_AEBSystem/ALG_AEBController/AEB_LongTgtInfoCalc/LongObjCalcInfoCalc/AEBS_LongCollisionAssesApp/collisionSeverityHighCalc/collisionSeverityHighCalc2/collisionSeverityHighPhase1/Phase1TimeCalc/TimeWorkPhase1Calc ==
  tMaxReduce : Inport
  tEgoStop : Inport  Port=2
  tBrakeLatency : Inport  Port=3
  MinMax : MinMax  Inputs=3
  tWorkPhase1 : Outport
  wires:
  tMaxReduce/1 -> MinMax/1
  tEgoStop/1 -> MinMax/2
  MinMax/1 -> tWorkPhase1/1
  tBrakeLatency/1 -> MinMax/3

== system TosAeb/FcwAebJa_Step_sys/ALG_AEBSystem/ALG_AEBController/AEB_LongTgtInfoCalc/LongObjCalcInfoCalc/AEBS_LongCollisionAssesApp/collisionSeverityHighCalc/collisionSeverityHighCalc2/collisionSeverityHighPhase1/Phase1TimeCalc/tEgoStopWorkPhase1 ==
  note: if subjAccel < 0
      | tEgoStop = subjVel/(-subjAccel);
      | else
      | tEgoStop = inf;
  subjLongAccel : Inport
  subjVel : Inport  Port=2
  If1 : If  IfExpression=u1 < 0
  Merge1 : Merge
  TimeEgoStopCalc : Subsystem  TreatAsAtomicUnit=on
  TimeEgoStopInf : Subsystem  TreatAsAtomicUnit=on
  tEgoStop : Outport
  wires:
  subjVel/1 -> TimeEgoStopCalc/2(subjVel)
  [EGONOSTOP] -> TimeEgoStopInf/Action
  TimeEgoStopInf/1(tEgoStop) -> Merge1/2
  Merge1/1 -> tEgoStop/1  "tEgoStop"
  subjLongAccel/1 -> TimeEgoStopCalc/1(subjLongAccel), If1/1
  If1/2 -> [EGONOSTOP]
  TimeEgoStopCalc/1(tEgoStop) -> Merge1/1
  If1/1 -> TimeEgoStopCalc/Action

== system TosAeb/FcwAebJa_Step_sys/ALG_AEBSystem/ALG_AEBController/AEB_LongTgtInfoCalc/LongObjCalcInfoCalc/AEBS_LongCollisionAssesApp/collisionSeverityHighCalc/collisionSeverityHighCalc2/collisionSeverityHighPhase1/Phase1TimeCalc/tMaxReducePhase1Calc ==
  note: if subjAccel < 0
      | calculate tMaxReduce;
      | else
      | tMaxReduced = inf;
  subjLongAccel : Inport
  velMaxReudce : Inport  Port=2
  subjVel : Inport  Port=3
  If : If  IfExpression=u1 < 0
  Merge : Merge
  TimeMaxReduceCalc : Subsystem  TreatAsAtomicUnit=on
  TimeMaxReduceInf : Subsystem  TreatAsAtomicUnit=on
  tMaxReduce : Outport
  wires:
  velMaxReudce/1 -> TimeMaxReduceCalc/2(velMaxReudce)
  TimeMaxReduceInf/1(tMaxReduce) -> Merge/2
  subjLongAccel/1 -> If/1, TimeMaxReduceCalc/1(subjLongAccel)
  subjVel/1 -> TimeMaxReduceCalc/3(subjVel)
  Merge/1 -> tMaxReduce/1  "tMaxReduce"
  If/2 -> [TINF]
  [TINF] -> TimeMaxReduceInf/Action
  TimeMaxReduceCalc/1(tMaxReduce) -> Merge/1
  If/1 -> TimeMaxReduceCalc/Action

== system TosAeb/FcwAebJa_Step_sys/ALG_AEBSystem/ALG_AEBController/AEB_LongTgtInfoCalc/LongObjCalcInfoCalc/AEBS_LongCollisionAssesApp/collisionSeverityHighCalc/collisionSeverityHighCalc2/collisionSeverityHighPhase1/Phase1TimeCalc/tObjStopCalc ==
  accelAbs : Inport
  velAbs : Inport  Port=2
  StopTimeFlow : Stateflow chart  TreatAsAtomicUnit=on
  tStop : Outport
  wires:
  velAbs/1 -> StopTimeFlow/2(velRel)  "velAbs"
  accelAbs/1 -> StopTimeFlow/1(accelRel)  "accelAbs"
  StopTimeFlow/1(tStop) -> tStop/1  "tStop"

== system TosAeb/FcwAebJa_Step_sys/ALG_AEBSystem/ALG_AEBController/AEB_LongTgtInfoCalc/LongObjCalcInfoCalc/AEBS_LongCollisionAssesApp/collisionSeverityHighCalc/collisionSeverityHighCalc2/collisionSeverityHighPhase2/Phase2TimeCalc/TimeEgoStopCalc ==
  note: if subjVelWorkPhase1 < 0
      | 	Calculate tEgoStop;
      | else
      | 	tEgoStop = +inf;
  subjVelWorkPhase1 : Inport
  subjLongAccelWorkPhase1 : Inport  Port=2
  If1 : If  IfExpression=u1 < 0
  Merge1 : Merge
  TimeEgoStopCalc : Subsystem  TreatAsAtomicUnit=on
  TimeEgoStopInf : Subsystem  TreatAsAtomicUnit=on
  tEgoStop : Outport
  wires:
  subjVelWorkPhase1/1 -> TimeEgoStopCalc/1(subjVelWorkPhase1)
  subjLongAccelWorkPhase1/1 -> If1/1, TimeEgoStopCalc/2(subjLongAccelWorkPhase1)
  TimeEgoStopInf/1(tEgoStop) -> Merge1/2
  If1/1 -> TimeEgoStopCalc/Action
  TimeEgoStopCalc/1(tEgoStop) -> Merge1/1
  Merge1/1 -> tEgoStop/1  "tEgoStop"
  If1/2 -> [TimeEgoInf]
  [TimeEgoInf] -> TimeEgoStopInf/Action

== system TosAeb/FcwAebJa_Step_sys/ALG_AEBSystem/ALG_AEBController/AEB_LongTgtInfoCalc/LongObjCalcInfoCalc/AEBS_LongCollisionAssesApp/collisionSeverityHighCalc/collisionSeverityHighCalc2/collisionSeverityHighPhase2/Phase2TimeCalc/TimeGradientPhase2Calc ==
  note: if subjAccelWorkPhase1 > accelMaxReduce
      | 	Calculate tGradientPhase2
      | else
      | 	tGradientPhase2 = 0;
  subjLongAccelWorkPhase1 : Inport
  accelMaxReudce : Inport  Port=2
  accelGradient : Inport  Port=3
  If Action Subsystem6 : Subsystem  TreatAsAtomicUnit=on
  If Action Subsystem7 : Subsystem  TreatAsAtomicUnit=on
  If3 : If  NumInputs=2  IfExpression=u1>u2
  Merge3 : Merge
  tGradientPhase2 : Outport
  wires:
  Merge3/1 -> tGradientPhase2/1  "tGradientPhase2"
  If3/1 -> If Action Subsystem7/Action
  If Action Subsystem6/1(tGradientPhase2) -> Merge3/2
  If Action Subsystem7/1(tGradientPhase2) -> Merge3/1
  If3/2 -> [TimeGradientNull]
  [TimeGradientNull] -> If Action Subsystem6/Action
  accelGradient/1 -> If Action Subsystem7/3(accelGradient)
  accelMaxReudce/1 -> If Action Subsystem7/1(accelMaxReudce), If3/2
  subjLongAccelWorkPhase1/1 -> If Action Subsystem7/2(subjLongAccelWorkPhase1), If3/1

== system TosAeb/FcwAebJa_Step_sys/ALG_AEBSystem/ALG_AEBController/AEB_LongTgtInfoCalc/LongObjCalcInfoCalc/AEBS_LongCollisionAssesApp/collisionSeverityHighCalc/collisionSeverityHighCalc2/collisionSeverityHighPhase2/Phase2TimeCalc/TimeWorkPhase2Calc ==
  tMaxReduce : Inport
  tEgoStop : Inport  Port=2
  tGradientPhase2 : Inport  Port=3
  MinMax : MinMax  Inputs=3
  tWorkPhase2 : Outport
  wires:
  tEgoStop/1 -> MinMax/2
  tMaxReduce/1 -> MinMax/1
  MinMax/1 -> tWorkPhase2/1
  tGradientPhase2/1 -> MinMax/3

== system TosAeb/FcwAebJa_Step_sys/ALG_AEBSystem/ALG_AEBController/AEB_LongTgtInfoCalc/LongObjCalcInfoCalc/AEBS_LongCollisionAssesApp/collisionSeverityHighCalc/collisionSeverityHighCalc2/collisionSeverityHighPhase2/Phase2TimeCalc/tMaxReducePhase2Calc ==
  note: if subjAccel < 0
      | 	Calculate tMaxReduced;
      | else
      | 	tMaxReduced = inf;
  subjLongAccelWorkPhase1 : Inport
  velMaxReudce : Inport  Port=2
  subjVelWorkPhase1 : Inport  Port=3
  If : If  IfExpression=u1 < 0
  Merge : Merge
  TimeMaxReduceCalc : Subsystem  TreatAsAtomicUnit=on
  TimeMaxReduceInf : Subsystem  TreatAsAtomicUnit=on
  tMaxReduce : Outport
  wires:
  TimeMaxReduceInf/1(tMaxReduce) -> Merge/2
  If/1 -> TimeMaxReduceCalc/Action
  TimeMaxReduceCalc/1(tMaxReduce) -> Merge/1
  Merge/1 -> tMaxReduce/1  "tMaxReduce"
  If/2 -> [TIMEMAXINF]
  [TIMEMAXINF] -> TimeMaxReduceInf/Action
  subjLongAccelWorkPhase1/1 -> TimeMaxReduceCalc/1(subjLongAccelWorkPhase1), If/1
  subjVelWorkPhase1/1 -> TimeMaxReduceCalc/3(subjVelWorkPhase1)
  velMaxReudce/1 -> TimeMaxReduceCalc/2(velMaxReudce)

== system TosAeb/FcwAebJa_Step_sys/ALG_AEBSystem/ALG_AEBController/AEB_LongTgtInfoCalc/LongObjCalcInfoCalc/AEBS_LongCollisionAssesApp/collisionSeverityHighCalc/collisionSeverityHighCalc2/collisionSeverityHighPhase2/Phase2TimeCalc/tObjStopCalc ==
  accelAbs : Inport
  velAbs : Inport  Port=2
  StopTimeFlow : Stateflow chart  TreatAsAtomicUnit=on
  tStop : Outport
  wires:
  velAbs/1 -> StopTimeFlow/2(velRel)  "velAbs"
  accelAbs/1 -> StopTimeFlow/1(accelRel)  "accelAbs"
  StopTimeFlow/1(tStop) -> tStop/1  "tStop"

== system TosAeb/FcwAebJa_Step_sys/ALG_AEBSystem/ALG_AEBController/AEB_LongTgtInfoCalc/LongObjCalcInfoCalc/AEBS_LongCollisionAssesApp/collisionSeverityHighCalc/collisionSeverityHighCalc2/collisionSeverityHighPhase3/Phase3TimeCalc/TimeEgoStopCalc ==
  note: if subjAccelWorkPhase2 < 0
      | 	Calculate tEgoStop;
      | else
      | 	tEgoStop = +inf;
  accelMaxReduce : Inport
  subjVelWorkPhase2 : Inport  Port=2
  If1 : If  IfExpression=u1 < 0
  Merge1 : Merge
  TimeEgoStopCalc : Subsystem  TreatAsAtomicUnit=on
  TimeEgoStopInf : Subsystem  TreatAsAtomicUnit=on
  tEgoStop : Outport
  wires:
  TimeEgoStopInf/1(tEgoStop) -> Merge1/2
  TimeEgoStopCalc/1(tEgoStop) -> Merge1/1
  If1/1 -> TimeEgoStopCalc/Action
  accelMaxReduce/1 -> TimeEgoStopCalc/1(accelMaxReduce), If1/1
  subjVelWorkPhase2/1 -> TimeEgoStopCalc/2(subjVelWorkPhase2)
  If1/2 -> [TIMEEGOINF]
  [TIMEEGOINF] -> TimeEgoStopInf/Action
  Merge1/1 -> tEgoStop/1  "tEgoStop"

== system TosAeb/FcwAebJa_Step_sys/ALG_AEBSystem/ALG_AEBController/AEB_LongTgtInfoCalc/LongObjCalcInfoCalc/AEBS_LongCollisionAssesApp/collisionSeverityHighCalc/collisionSeverityHighCalc2/collisionSeverityHighPhase3/Phase3TimeCalc/TimeWorkPhase3Calc ==
  tMaxReduce : Inport
  tEgoStop : Inport  Port=2
  tBrakeConst : Inport  Port=3
  MinMax : MinMax  Inputs=3
  tWorkPhase3 : Outport
  wires:
  tEgoStop/1 -> MinMax/2
  MinMax/1 -> tWorkPhase3/1
  tMaxReduce/1 -> MinMax/1
  tBrakeConst/1 -> MinMax/3

== system TosAeb/FcwAebJa_Step_sys/ALG_AEBSystem/ALG_AEBController/AEB_LongTgtInfoCalc/LongObjCalcInfoCalc/AEBS_LongCollisionAssesApp/collisionSeverityHighCalc/collisionSeverityHighCalc2/collisionSeverityHighPhase3/Phase3TimeCalc/tBrakeConstCalc ==
  subjVelWorkPhase2 : Inport
  vxAbsWorkPhase2Target : Inport  Port=2
  accelMaxReduce : Inport  Port=3
  axAbsWorkPhase2Target : Inport  Port=4
  Bus Creator : BusCreator  Inputs=4
  Bus Selector : BusSelector  OutputSignals=accelMaxReduce,subjVelWorkPhase2,vxAbsWorkPhase2Target,axAbsWorkPhase2Target
  Bus Selector1 : BusSelector  OutputSignals=subjVelWorkPhase2,accelMaxReduce
  Bus Selector2 : BusSelector  OutputSignals=subjVelWorkPhase2,vxAbsWorkPhase2Target,accelMaxReduce,axAbsWorkPhase2Target
  tBrakeConstCalcFlow : Stateflow chart  TreatAsAtomicUnit=on
  tBrakeConstCalcSituation1 : Subsystem  TreatAsAtomicUnit=on
  tBrakeConstCalcSituation2 : Subsystem  TreatAsAtomicUnit=on
  tBrakeConst : Outport
  wires:
  tBrakeConstCalcSituation2/1(tBrakeConst) -> tBrakeConstCalcFlow/6(tBrakeConstIn2)
  tBrakeConstCalcSituation1/1(tBrakeConst) -> tBrakeConstCalcFlow/5(tBrakeConstIn1)
  tBrakeConstCalcFlow/2(Stuaiton1()) -> tBrakeConstCalcSituation1/Trigger
  tBrakeConstCalcFlow/3(Stuaiton2()) -> [Situaiton2]
  [Situaiton2] -> tBrakeConstCalcSituation2/Trigger
  tBrakeConstCalcFlow/1(tBrakeConst) -> tBrakeConst/1  "tBrakeConst"
  axAbsWorkPhase2Target/1 -> Bus Creator/4  "axAbsWorkPhase2Target"
  accelMaxReduce/1 -> Bus Creator/3  "accelMaxReduce"
  vxAbsWorkPhase2Target/1 -> Bus Creator/2  "vxAbsWorkPhase2Target"
  subjVelWorkPhase2/1 -> Bus Creator/1  "subjVelWorkPhase2"
  Bus Creator/1 -> Bus Selector/1, Bus Selector1/1, Bus Selector2/1
  Bus Selector/3 -> tBrakeConstCalcFlow/3(vxAbsWorkPhase2Target)  "<vxAbsWorkPhase2Target>"
  Bus Selector/2 -> tBrakeConstCalcFlow/2(subjVelWorkPhase2)  "<subjVelWorkPhase2>"
  Bus Selector/4 -> tBrakeConstCalcFlow/4(axAbsWorkPhase2Target)  "<axAbsWorkPhase2Target>"
  Bus Selector/1 -> tBrakeConstCalcFlow/1(accelMaxReduce)  "<accelMaxReduce>"
  Bus Selector1/1 -> tBrakeConstCalcSituation1/1(subjVelWorkPhase2)  "<subjVelWorkPhase2>"
  Bus Selector1/2 -> tBrakeConstCalcSituation1/2(accelMaxReduce)  "<accelMaxReduce>"
  Bus Selector2/3 -> tBrakeConstCalcSituation2/3(accelMaxReduce)  "<accelMaxReduce>"
  Bus Selector2/2 -> tBrakeConstCalcSituation2/2(vxAbsWorkPhase2Target)  "<vxAbsWorkPhase2Target>"
  Bus Selector2/4 -> tBrakeConstCalcSituation2/4(axAbsWorkPhase2Target)  "<axAbsWorkPhase2Target>"
  Bus Selector2/1 -> tBrakeConstCalcSituation2/1(subjVelWorkPhase2)  "<subjVelWorkPhase2>"
